simulink model "V2x_TC_RoadWorksWarning_cs"  (Simulink 7.6)
settings: Solver=ode5  SolverMode=SingleTasking  StartTime=0.0  StopTime=22  FixedStep=1/100  RelTol=1e-3
PreLoadFcn:
  | check_NUTS_defined;
  | 
  | assignin('base',['model_closed_' bdroot],false);addpath('../includes_V2x');
InitFcn:
  | PreScanExperimentInitFcn(bdroot)
StopFcn:
  | PreScanExperimentStopFcn(bdroot)
undefined symbols (not defined in the file or in its folder): acceleration, actorID, actorType, DiscIntNeverNeededParam, GPS_alt, GPS_lat, GPS_long, intmax, lightBarInUse, messageID, Newton, publicActorType, Raphson, rotZ, sireneInUse, trafficLightPriority, turnAdvice, velocity, x_ini_VRML, y_ini_VRML, yawRate, z_ini_VRML
libraries: simulink x62, PreScanSimulinkLibrary x45, PreScanUsefulBlocks x20, simulink_extras x1
(part 5 of 15)

== system V2x_TC_RoadWorksWarning_cs/STATE_Toyota_Yaris_1 ==
  State : Inport  SampleTime=TimeStep
  Acceleration : Subsystem  mask(ToSensorWorld){PortName=Acceleration_Toyota_Yaris_1, PortIndex=499, PortWidth=3, TimeStep=1/100}
      mask prompts: PortName "Port Name [-]"; PortIndex "Index [-]"; PortWidth "Port Dimensions [-]"; TimeStep "Upload Period [s]"
      mask help: Sends signal(s) from Simulink to the Sensor World
      mask Help:
      | Sends signal(s) from Simulink to the Sensor World.
      | 
      | - Port Name [-]: specifies the name of the output terminal of the Simulink block.
      | - Index [-]: specifies the start position in the output vector sent to the Sensor World
      | - Port dimensions [-]: specifies the width (number of scalars) of the output port
      | - Upload period [s]: specifies the upload period (in seconds) of the port.
      mask Display: port_label('input', 1, PortName)
  Demux : Demux  Outputs=5
  Position : Subsystem  mask(ToSensorWorld){PortName=Position_Toyota_Yaris_1, PortIndex=493, PortWidth=3, TimeStep=1/100}
      mask prompts: PortName "Port Name [-]"; PortIndex "Index [-]"; PortWidth "Port Dimensions [-]"; TimeStep "Upload Period [s]"
      mask help: Sends signal(s) from Simulink to the Sensor World
      mask Help:
      | Sends signal(s) from Simulink to the Sensor World.
      | 
      | - Port Name [-]: specifies the name of the output terminal of the Simulink block.
      | - Index [-]: specifies the start position in the output vector sent to the Sensor World
      | - Port dimensions [-]: specifies the width (number of scalars) of the output port
      | - Upload period [s]: specifies the upload period (in seconds) of the port.
      mask Display: port_label('input', 1, PortName)
  RPY : Subsystem  mask(ToSensorWorld){PortName=RPY_Toyota_Yaris_1, PortIndex=502, PortWidth=3, TimeStep=1/100}
      mask prompts: PortName "Port Name [-]"; PortIndex "Index [-]"; PortWidth "Port Dimensions [-]"; TimeStep "Upload Period [s]"
      mask help: Sends signal(s) from Simulink to the Sensor World
      mask Help:
      | Sends signal(s) from Simulink to the Sensor World.
      | 
      | - Port Name [-]: specifies the name of the output terminal of the Simulink block.
      | - Index [-]: specifies the start position in the output vector sent to the Sensor World
      | - Port dimensions [-]: specifies the width (number of scalars) of the output port
      | - Upload period [s]: specifies the upload period (in seconds) of the port.
      mask Display: port_label('input', 1, PortName)
  Velocity : Subsystem  mask(ToSensorWorld){PortName=Velocity_Toyota_Yaris_1, PortIndex=496, PortWidth=3, TimeStep=1/100}
      mask prompts: PortName "Port Name [-]"; PortIndex "Index [-]"; PortWidth "Port Dimensions [-]"; TimeStep "Upload Period [s]"
      mask help: Sends signal(s) from Simulink to the Sensor World
      mask Help:
      | Sends signal(s) from Simulink to the Sensor World.
      | 
      | - Port Name [-]: specifies the name of the output terminal of the Simulink block.
      | - Index [-]: specifies the start position in the output vector sent to the Sensor World
      | - Port dimensions [-]: specifies the width (number of scalars) of the output port
      | - Upload period [s]: specifies the upload period (in seconds) of the port.
      mask Display: port_label('input', 1, PortName)
  iniBODYAngles wrtVEH [rad] : Constant  Value=[Roll0 Pitch0 0]  SampleTime=TimeStep
  iniVEHAngles wrtGLOB [rad] : Constant  Value=[0 0 Yaw0]  SampleTime=TimeStep
  iniVEHposition wrtGLOB [m] : Constant  Value=[x0 y0 z0]  SampleTime=TimeStep
  state2FullState : PreScanSimulinkLibrary/state2FullState  SystemSampleTime=-1
  velRPY : Subsystem  mask(ToSensorWorld){PortName=velRPY_Toyota_Yaris_1, PortIndex=505, PortWidth=3, TimeStep=1/100}
      mask prompts: PortName "Port Name [-]"; PortIndex "Index [-]"; PortWidth "Port Dimensions [-]"; TimeStep "Upload Period [s]"
      mask help: Sends signal(s) from Simulink to the Sensor World
      mask Help:
      | Sends signal(s) from Simulink to the Sensor World.
      | 
      | - Port Name [-]: specifies the name of the output terminal of the Simulink block.
      | - Index [-]: specifies the start position in the output vector sent to the Sensor World
      | - Port dimensions [-]: specifies the width (number of scalars) of the output port
      | - Upload period [s]: specifies the upload period (in seconds) of the port.
      mask Display: port_label('input', 1, PortName)
  wires:
  Demux/5 -> velRPY/1(Y)
  Demux/4 -> RPY/1(Y)
  Demux/3 -> Acceleration/1(Y)
  Demux/2 -> Velocity/1(Y)
  Demux/1 -> Position/1(Y)
  State/1 -> state2FullState/1
  state2FullState/1 -> Demux/1
  iniVEHposition wrtGLOB [m]/1 -> state2FullState/2
  iniBODYAngles wrtVEH [rad]/1 -> state2FullState/3
  iniVEHAngles wrtGLOB [rad]/1 -> state2FullState/4

== system V2x_TC_RoadWorksWarning_cs/SetActuators ==
  Bus Creator : BusCreator  Inputs=1
  Data Store Read : DataStoreRead  DataStoreName=SimCoreActuators  SampleTime=TimeStep
  SendActuators : M-S-Function  FunctionName=SendActuatorsBlock  Parameters=TimeStep NrOfActuators
  dummy : Outport
  tag scope: SimCoreInfo=global
  wires:
  Bus Creator/1 -> [SimCoreInfo]  "SimCore"
  SendActuators/2 -> Bus Creator/1  "ComputationTime[s]"
  SendActuators/1 -> dummy/1
  Data Store Read/1 -> SendActuators/1

== system V2x_TC_RoadWorksWarning_cs/SimCoreInfo ==
  Bus Selector : BusSelector  OutputSignals=ComputationTime[s]
  Time : Scope
      settings: TimeRange=10  YMin=0  YMax=0.5  SaveName=ComputationTime
  wires:
  Bus Selector/1 -> Time/1  "<ComputationTime[s]>"
  [SimCoreInfo] -> Bus Selector/1

== system V2x_TC_RoadWorksWarning_cs/Sphere_1 ==
  SELF_Sphere_1 : Inport  Tag=SELFInport
  Dynamics_Empty : Subsystem  Tag=Dynamics_Empty
      mask Display: port_label('output', 1, 'State');text(0,0,' Empty\n')
  Gain : Gain  Gain=[1 1 1 1 1 1 0 0 1]
  SELF_Demux : Subsystem  Tag=DemuxSELF
      settings: DeleteFcn=PreScanBlockDeleteFcn;
      mask Type: PreScan Demux Block:
      mask Display:
      | port_label('output',1,'x [m]')
      | port_label('output',2,'y [m]')
      | port_label('output',3,'z [m]','texmode','on')
      | port_label('output',4,'Rotx [deg]','texmode','on')
      | port_label('output',5,'Roty [deg]','texmode','on')
      | port_label('output',6,'Rotz [deg]','texmode','on')
      | port_label('output',7,'GPS lat [deg]','texmode','on')
      | port_label('output',8,'GPS long [deg]','texmode','on')
      | port_label('output',9,'GPS alt [m]','texmode','on')
      | port_label('output',10,'Velocity [m/s]')
      | port_label('output',11,'Heading [deg]')
      | port_label('output',12,'Yaw rate [deg/s]')
  Terminator_{1..13} (13 alike) : Terminator
  STATE_Sphere_1 : Outport  Tag=StateOutport
  wires:
  [state] -> Gain/1
  SELF_Sphere_1/1 -> SELF_Demux/1(SELF)
  Gain/1 -> STATE_Sphere_1/1
  Dynamics_Empty/1(State) -> Terminator_1/1
  SELF_Demux/1(x [m]) -> Terminator_2/1
  SELF_Demux/2(y [m]) -> Terminator_3/1
  SELF_Demux/3(z [m]) -> Terminator_4/1
  SELF_Demux/4(rotx [deg]) -> Terminator_5/1
  SELF_Demux/5(roty [deg]) -> Terminator_6/1
  SELF_Demux/6(rotz [deg]) -> Terminator_7/1
  SELF_Demux/7(GPS lat [deg]) -> Terminator_8/1
  SELF_Demux/8(GPS long [deg]) -> Terminator_9/1
  SELF_Demux/9(GPS alt [m]) -> Terminator_10/1
  SELF_Demux/10(velocity [m/s]) -> Terminator_11/1
  SELF_Demux/11(heading [deg]) -> Terminator_12/1
  SELF_Demux/12(yaw rate [deg/s]) -> Terminator_13/1

== system V2x_TC_RoadWorksWarning_cs/Toyota_Previa_1 ==
  SELF_Toyota_Previa_1 : Inport  Tag=SELFInport
  Dynamics_Empty : Subsystem  Tag=Dynamics_Empty
      mask Display: port_label('output', 1, 'State');text(0,0,' Empty\n')
  SELF_Demux : Subsystem  Tag=DemuxSELF
      settings: DeleteFcn=PreScanBlockDeleteFcn;
      mask Type: PreScan Demux Block:
      mask Display:
      | port_label('output',1,'x [m]')
      | port_label('output',2,'y [m]')
      | port_label('output',3,'z [m]','texmode','on')
      | port_label('output',4,'Rotx [deg]','texmode','on')
      | port_label('output',5,'Roty [deg]','texmode','on')
      | port_label('output',6,'Rotz [deg]','texmode','on')
      | port_label('output',7,'GPS lat [deg]','texmode','on')
      | port_label('output',8,'GPS long [deg]','texmode','on')
      | port_label('output',9,'GPS alt [m]','texmode','on')
      | port_label('output',10,'Velocity [m/s]')
      | port_label('output',11,'Heading [deg]')
      | port_label('output',12,'Yaw rate [deg/s]')
  Terminator_{1..12} (12 alike) : Terminator
  STATE_Toyota_Previa_1 : Outport  Tag=StateOutport
  wires:
  SELF_Toyota_Previa_1/1 -> SELF_Demux/1(SELF)
  Dynamics_Empty/1(State) -> STATE_Toyota_Previa_1/1
  SELF_Demux/1(x [m]) -> Terminator_1/1
  SELF_Demux/2(y [m]) -> Terminator_2/1
  SELF_Demux/3(z [m]) -> Terminator_3/1
  SELF_Demux/4(rotx [deg]) -> Terminator_4/1
  SELF_Demux/5(roty [deg]) -> Terminator_5/1
  SELF_Demux/6(rotz [deg]) -> Terminator_6/1
  SELF_Demux/7(GPS lat [deg]) -> Terminator_7/1
  SELF_Demux/8(GPS long [deg]) -> Terminator_8/1
  SELF_Demux/9(GPS alt [m]) -> Terminator_9/1
  SELF_Demux/10(velocity [m/s]) -> Terminator_10/1
  SELF_Demux/11(heading [deg]) -> Terminator_11/1
  SELF_Demux/12(yaw rate [deg/s]) -> Terminator_12/1

== system V2x_TC_RoadWorksWarning_cs/Toyota_Yaris_1 ==
  SELF_Toyota_Yaris_1 : Inport  Tag=SELFInport
  Dynamics_Empty : Subsystem  Tag=Dynamics_Empty
      mask Display: port_label('output', 1, 'State');text(0,0,' Empty\n')
  SELF_Demux : Subsystem  Tag=DemuxSELF
      settings: DeleteFcn=PreScanBlockDeleteFcn;
      mask Type: PreScan Demux Block:
      mask Display:
      | port_label('output',1,'x [m]')
      | port_label('output',2,'y [m]')
      | port_label('output',3,'z [m]','texmode','on')
      | port_label('output',4,'Rotx [deg]','texmode','on')
      | port_label('output',5,'Roty [deg]','texmode','on')
      | port_label('output',6,'Rotz [deg]','texmode','on')
      | port_label('output',7,'GPS lat [deg]','texmode','on')
      | port_label('output',8,'GPS long [deg]','texmode','on')
      | port_label('output',9,'GPS alt [m]','texmode','on')
      | port_label('output',10,'Velocity [m/s]')
      | port_label('output',11,'Heading [deg]')
      | port_label('output',12,'Yaw rate [deg/s]')
  Terminator_{1..12} (12 alike) : Terminator
  STATE_Toyota_Yaris_1 : Outport  Tag=StateOutport
  wires:
  SELF_Toyota_Yaris_1/1 -> SELF_Demux/1(SELF)
  Dynamics_Empty/1(State) -> STATE_Toyota_Yaris_1/1
  SELF_Demux/1(x [m]) -> Terminator_1/1
  SELF_Demux/2(y [m]) -> Terminator_2/1
  SELF_Demux/3(z [m]) -> Terminator_3/1
  SELF_Demux/4(rotx [deg]) -> Terminator_4/1
  SELF_Demux/5(roty [deg]) -> Terminator_5/1
  SELF_Demux/6(rotz [deg]) -> Terminator_6/1
  SELF_Demux/7(GPS lat [deg]) -> Terminator_7/1
  SELF_Demux/8(GPS long [deg]) -> Terminator_8/1
  SELF_Demux/9(GPS alt [m]) -> Terminator_9/1
  SELF_Demux/10(velocity [m/s]) -> Terminator_10/1
  SELF_Demux/11(heading [deg]) -> Terminator_11/1
  SELF_Demux/12(yaw rate [deg/s]) -> Terminator_12/1

== system V2x_TC_RoadWorksWarning_cs/TrafficSignPoleD_1 ==
  Antenna_Tx_6_BusAssignment : BusAssignment  Tag=BusAntennaT  AssignedSignals=warning,messageID,actorID,actorType,trafficLightPriority,GPS_lat,GPS_long,GPS_alt,rotZ
  Antenna_Tx_6_Mux : Subsystem  Tag=MuxAntennaT  mask(PreScan Mux Block:){Channel=1, TimeStep=1/100}
      mask prompts: TimeStep "Time Step [s]"
      settings: DeleteFcn=PreScanBlockDeleteFcn;
      mask Display:
      | fprintf('Channel: %d',Channel)
      | port_label('input', 1, 'Warning signal ID')
      | port_label('input', 2, 'Maneuvre ID')
      | port_label('input', 3, 'Vehicle Type')
      | port_label('input', 4, 'Braking Capacity')
      | port_label('input', 5, 'Vehicle Size')
      | port_label('input', 6, 'spare')
  CAM generation time step : Constant  Value=0.1
  CAM repetition time step : Constant  Value=0.05
  Cartesian to GPS : Subsystem
  Green light : Constant  Value=0
  Ground_{1..5} (5 alike) : Ground
  Infrastructure CAM generator : Subsystem
  Orange light : Constant  Value=0
  Red light : Constant  Value=0
  X : Constant  Value=200
  Y : Constant  Value=-6
  Z : Constant  Value=0
  actorID : Constant  Value=9
  actorType : Constant  Value=14
  messageID : Constant  Value=2
  rotZ : Constant  Value=180
  warning : Constant  Value=0
  Antenna_Tx_6 : Outport  Tag=AntennaTOutport
  wires:
  CAM generation time step/1 -> Infrastructure CAM generator/1(CAM gen time step)
  CAM repetition time step/1 -> Infrastructure CAM generator/2(CAM rep time step)
  warning/1 -> Infrastructure CAM generator/3(warning)
  messageID/1 -> Infrastructure CAM generator/4(messageID)
  actorID/1 -> Infrastructure CAM generator/5(actorID)
  actorType/1 -> Infrastructure CAM generator/6(actorType)
  Red light/1 -> Infrastructure CAM generator/7(Red light)
  Orange light/1 -> Infrastructure CAM generator/8(Orange light)
  Green light/1 -> Infrastructure CAM generator/9(Green light)
  rotZ/1 -> Infrastructure CAM generator/13(rotZ)
  Cartesian to GPS/1(lat) -> Infrastructure CAM generator/10(GPS_lat)
  Cartesian to GPS/2(long) -> Infrastructure CAM generator/11(GPS_long)
  Cartesian to GPS/3(alt) -> Infrastructure CAM generator/12(GPS_alt)
  X/1 -> Cartesian to GPS/1(X)
  Y/1 -> Cartesian to GPS/2(Y)
  Z/1 -> Cartesian to GPS/3(Z)
  Antenna_Tx_6_Mux/2(Out2) -> [Antenna_Tx_6]
  [Antenna_Tx_6] -> Antenna_Tx_6_BusAssignment/1
  Antenna_Tx_6_BusAssignment/1 -> Antenna_Tx_6_Mux/6(spare)
  Antenna_Tx_6_Mux/1(Out1) -> Antenna_Tx_6/1
  Infrastructure CAM generator/9(CAM_rotZ) -> Antenna_Tx_6_BusAssignment/10
  Infrastructure CAM generator/8(CAM_GPSalt) -> Antenna_Tx_6_BusAssignment/9
  Infrastructure CAM generator/7(CAM_GPSlong) -> Antenna_Tx_6_BusAssignment/8
  Infrastructure CAM generator/6(CAM_GPSlat) -> Antenna_Tx_6_BusAssignment/7
  Infrastructure CAM generator/5(CAM_trafficLightPriority) -> Antenna_Tx_6_BusAssignment/6
  Infrastructure CAM generator/4(CAM_vehicleType) -> Antenna_Tx_6_BusAssignment/5
  Infrastructure CAM generator/3(CAM_vehicleID) -> Antenna_Tx_6_BusAssignment/4
  Infrastructure CAM generator/2(CAM_messageID) -> Antenna_Tx_6_BusAssignment/3
  Infrastructure CAM generator/1(CAM_warning) -> Antenna_Tx_6_BusAssignment/2
  Ground_1/1 -> Antenna_Tx_6_Mux/1(warning_signal_id)
  Ground_2/1 -> Antenna_Tx_6_Mux/2(maneuvre_id)
  Ground_3/1 -> Antenna_Tx_6_Mux/3(vehicle_type)
  Ground_4/1 -> Antenna_Tx_6_Mux/4(brakingcapacity)
  Ground_5/1 -> Antenna_Tx_6_Mux/5(vehicle_size)

== system V2x_TC_RoadWorksWarning_cs/TrafficSignPoleD_3 ==
  Antenna_Tx_4_BusAssignment : BusAssignment  Tag=BusAntennaT  AssignedSignals=actorID,warning,actorType,lightBarInUse,sireneInUse,trafficLightPriority,GPS_lat,GPS_long,GPS_alt
  Antenna_Tx_4_Mux : Subsystem  Tag=MuxAntennaT  mask(PreScan Mux Block:){Channel=1, TimeStep=1/100}
      mask prompts: TimeStep "Time Step [s]"
      settings: DeleteFcn=PreScanBlockDeleteFcn;
      mask Display:
      | fprintf('Channel: %d',Channel)
      | port_label('input', 1, 'Warning signal ID')
      | port_label('input', 2, 'Maneuvre ID')
      | port_label('input', 3, 'Vehicle Type')
      | port_label('input', 4, 'Braking Capacity')
      | port_label('input', 5, 'Vehicle Size')
      | port_label('input', 6, 'spare')
  CAM generation time step : Constant  Value=0.1
  CAM repetition time step : Constant  Value=0.05
  Cartesian to GPS : Subsystem
  Ground_{1..5} (5 alike) : Ground
  ITS actorID : Constant  Value=161
  ITS actorType : Constant  Value=25
  Infrastructure CAM generator ITS road : Subsystem
  Red light : Constant  Value=0
  Red light1 : Constant  Value=0
  Red light2 : Constant  Value=0
  X : Constant  Value=220
  Y : Constant  Value=-6.5
  Z : Constant  Value=0
  actorType : Constant
  Antenna_Tx_4 : Outport  Tag=AntennaTOutport
  wires:
  CAM generation time step/1 -> Infrastructure CAM generator ITS road/1(CAM gen time step)
  CAM repetition time step/1 -> Infrastructure CAM generator ITS road/2(CAM rep time step)
  ITS actorID/1 -> Infrastructure CAM generator ITS road/3(ITS actorID)
  ITS actorType/1 -> Infrastructure CAM generator ITS road/4(ITS actorType)
  [transmission] -> Infrastructure CAM generator ITS road/5(warning)
  actorType/1 -> Infrastructure CAM generator ITS road/6(actorType)
  Red light/1 -> Infrastructure CAM generator ITS road/7(lightBarInUse)
  Red light1/1 -> Infrastructure CAM generator ITS road/9(trafficLightPriority)
  X/1 -> Cartesian to GPS/1(X)
  Y/1 -> Cartesian to GPS/2(Y)
  Z/1 -> Cartesian to GPS/3(Z)
  Red light2/1 -> Infrastructure CAM generator ITS road/8(sireneInUse)
  Cartesian to GPS/1(lat) -> Infrastructure CAM generator ITS road/10(GPS_lat)
  Cartesian to GPS/2(long) -> Infrastructure CAM generator ITS road/11(GPS_long)
  Cartesian to GPS/3(alt) -> Infrastructure CAM generator ITS road/12(GPS_alt)
  Antenna_Tx_4_Mux/2(Out2) -> [Antenna_Tx_4]
  [Antenna_Tx_4] -> Antenna_Tx_4_BusAssignment/1
  Antenna_Tx_4_BusAssignment/1 -> Antenna_Tx_4_Mux/6(spare)
  Antenna_Tx_4_Mux/1(Out1) -> Antenna_Tx_4/1
  Infrastructure CAM generator ITS road/1(CAM_actorID) -> Antenna_Tx_4_BusAssignment/2
  Infrastructure CAM generator ITS road/2(CAM_warning) -> Antenna_Tx_4_BusAssignment/3
  Infrastructure CAM generator ITS road/3(CAM_actorType) -> Antenna_Tx_4_BusAssignment/4
  Infrastructure CAM generator ITS road/4(CAM_lightBarInUse) -> Antenna_Tx_4_BusAssignment/5
  Infrastructure CAM generator ITS road/5(CAM_sireneInUse) -> Antenna_Tx_4_BusAssignment/6
  Infrastructure CAM generator ITS road/6(CAM_trafficLightPriority) -> Antenna_Tx_4_BusAssignment/7
  Infrastructure CAM generator ITS road/7(CAM_GPS_lat) -> Antenna_Tx_4_BusAssignment/8
  Infrastructure CAM generator ITS road/8(CAM_GPS_long) -> Antenna_Tx_4_BusAssignment/9
  Infrastructure CAM generator ITS road/9(CAM_GPS_alt) -> Antenna_Tx_4_BusAssignment/10
  Ground_1/1 -> Antenna_Tx_4_Mux/1(warning_signal_id)
  Ground_2/1 -> Antenna_Tx_4_Mux/2(maneuvre_id)
  Ground_3/1 -> Antenna_Tx_4_Mux/3(vehicle_type)
  Ground_4/1 -> Antenna_Tx_4_Mux/4(brakingcapacity)
  Ground_5/1 -> Antenna_Tx_4_Mux/5(vehicle_size)

== system V2x_TC_RoadWorksWarning_cs/TrafficSignPoleD_6 ==
  Antenna_Tx_5_BusAssignment : BusAssignment  Tag=BusAntennaT  AssignedSignals=warning,messageID,actorID,actorType,trafficLightPriority,GPS_lat,GPS_long,GPS_alt,rotZ
  Antenna_Tx_5_Mux : Subsystem  Tag=MuxAntennaT  mask(PreScan Mux Block:){Channel=1, TimeStep=1/100}
      mask prompts: TimeStep "Time Step [s]"
      settings: DeleteFcn=PreScanBlockDeleteFcn;
      mask Display:
      | fprintf('Channel: %d',Channel)
      | port_label('input', 1, 'Warning signal ID')
      | port_label('input', 2, 'Maneuvre ID')
      | port_label('input', 3, 'Vehicle Type')
      | port_label('input', 4, 'Braking Capacity')
      | port_label('input', 5, 'Vehicle Size')
      | port_label('input', 6, 'spare')
  CAM generation time step : Constant  Value=0.1
  CAM repetition time step : Constant  Value=0.05
  Cartesian to GPS : Subsystem
  Green light : Constant  Value=0
  Ground_{1..5} (5 alike) : Ground
  Infrastructure CAM generator : Subsystem
  Orange light : Constant  Value=0
  Red light : Constant  Value=0
  X : Constant  Value=110
  Y : Constant  Value=-6.5
  Z : Constant  Value=0
  actorID : Constant  Value=8
  actorType : Constant  Value=14
  messageID : Constant
  rotZ : Constant  Value=180
  warning : Constant  Value=0
  Antenna_Tx_5 : Outport  Tag=AntennaTOutport
  wires:
  CAM generation time step/1 -> Infrastructure CAM generator/1(CAM gen time step)
  CAM repetition time step/1 -> Infrastructure CAM generator/2(CAM rep time step)
  warning/1 -> Infrastructure CAM generator/3(warning)
  messageID/1 -> Infrastructure CAM generator/4(messageID)
  actorID/1 -> Infrastructure CAM generator/5(actorID)
  actorType/1 -> Infrastructure CAM generator/6(actorType)
  Red light/1 -> Infrastructure CAM generator/7(Red light)
  Orange light/1 -> Infrastructure CAM generator/8(Orange light)
  Green light/1 -> Infrastructure CAM generator/9(Green light)
  rotZ/1 -> Infrastructure CAM generator/13(rotZ)
  Cartesian to GPS/1(lat) -> Infrastructure CAM generator/10(GPS_lat)
  Cartesian to GPS/2(long) -> Infrastructure CAM generator/11(GPS_long)
  Cartesian to GPS/3(alt) -> Infrastructure CAM generator/12(GPS_alt)
  X/1 -> Cartesian to GPS/1(X)
  Y/1 -> Cartesian to GPS/2(Y)
  Z/1 -> Cartesian to GPS/3(Z)
  Antenna_Tx_5_Mux/2(Out2) -> [Antenna_Tx_5]
  [Antenna_Tx_5] -> Antenna_Tx_5_BusAssignment/1
  Antenna_Tx_5_BusAssignment/1 -> Antenna_Tx_5_Mux/6(spare)
  Antenna_Tx_5_Mux/1(Out1) -> Antenna_Tx_5/1
  Infrastructure CAM generator/9(CAM_rotZ) -> Antenna_Tx_5_BusAssignment/10
  Infrastructure CAM generator/8(CAM_GPSalt) -> Antenna_Tx_5_BusAssignment/9
  Infrastructure CAM generator/7(CAM_GPSlong) -> Antenna_Tx_5_BusAssignment/8
  Infrastructure CAM generator/6(CAM_GPSlat) -> Antenna_Tx_5_BusAssignment/7
  Infrastructure CAM generator/5(CAM_trafficLightPriority) -> Antenna_Tx_5_BusAssignment/6
  Infrastructure CAM generator/4(CAM_vehicleType) -> Antenna_Tx_5_BusAssignment/5
  Infrastructure CAM generator/3(CAM_vehicleID) -> Antenna_Tx_5_BusAssignment/4
  Infrastructure CAM generator/2(CAM_messageID) -> Antenna_Tx_5_BusAssignment/3
  Infrastructure CAM generator/1(CAM_warning) -> Antenna_Tx_5_BusAssignment/2
  Ground_1/1 -> Antenna_Tx_5_Mux/1(warning_signal_id)
  Ground_2/1 -> Antenna_Tx_5_Mux/2(maneuvre_id)
  Ground_3/1 -> Antenna_Tx_5_Mux/3(vehicle_type)
  Ground_4/1 -> Antenna_Tx_5_Mux/4(brakingcapacity)
  Ground_5/1 -> Antenna_Tx_5_Mux/5(vehicle_size)

== system V2x_TC_RoadWorksWarning_cs/TrafficSignPoleNL_1 ==
  Antenna_Tx_2_BusAssignment : BusAssignment  Tag=BusAntennaT  AssignedSignals=warning,messageID,actorID,actorType,trafficLightPriority,GPS_lat,GPS_long,GPS_alt,rotZ
  Antenna_Tx_2_Mux : Subsystem  Tag=MuxAntennaT  mask(PreScan Mux Block:){Channel=1, TimeStep=1/100}
      mask prompts: TimeStep "Time Step [s]"
      settings: DeleteFcn=PreScanBlockDeleteFcn;
      mask Display:
      | fprintf('Channel: %d',Channel)
      | port_label('input', 1, 'Warning signal ID')
      | port_label('input', 2, 'Maneuvre ID')
      | port_label('input', 3, 'Vehicle Type')
      | port_label('input', 4, 'Braking Capacity')
      | port_label('input', 5, 'Vehicle Size')
      | port_label('input', 6, 'spare')
  CAM generation time step : Constant  Value=0.1
  CAM repetition time step : Constant  Value=0.05
  Cartesian to GPS : Subsystem
  Green light : Constant  Value=0
  Ground_{1..5} (5 alike) : Ground
  Infrastructure CAM generator : Subsystem
  Orange light : Constant  Value=0
  Red light : Constant  Value=0
  X : Constant  Value=122
  Y : Constant  Value=-6
  Z : Constant  Value=0
  actorID : Constant  Value=7
  actorType : Constant  Value=14
  messageID : Constant  Value=1060
  rotZ : Constant  Value=180
  warning : Constant  Value=0
  Antenna_Tx_2 : Outport  Tag=AntennaTOutport
  wires:
  CAM generation time step/1 -> Infrastructure CAM generator/1(CAM gen time step)
  CAM repetition time step/1 -> Infrastructure CAM generator/2(CAM rep time step)
  warning/1 -> Infrastructure CAM generator/3(warning)
  messageID/1 -> Infrastructure CAM generator/4(messageID)
  actorID/1 -> Infrastructure CAM generator/5(actorID)
  actorType/1 -> Infrastructure CAM generator/6(actorType)
  Red light/1 -> Infrastructure CAM generator/7(Red light)
  Orange light/1 -> Infrastructure CAM generator/8(Orange light)
  Green light/1 -> Infrastructure CAM generator/9(Green light)
  rotZ/1 -> Infrastructure CAM generator/13(rotZ)
  Cartesian to GPS/1(lat) -> Infrastructure CAM generator/10(GPS_lat)
  Cartesian to GPS/2(long) -> Infrastructure CAM generator/11(GPS_long)
  Cartesian to GPS/3(alt) -> Infrastructure CAM generator/12(GPS_alt)
  X/1 -> Cartesian to GPS/1(X)
  Y/1 -> Cartesian to GPS/2(Y)
  Z/1 -> Cartesian to GPS/3(Z)
  Antenna_Tx_2_Mux/2(Out2) -> [Antenna_Tx_2]
  [Antenna_Tx_2] -> Antenna_Tx_2_BusAssignment/1
  Antenna_Tx_2_BusAssignment/1 -> Antenna_Tx_2_Mux/6(spare)
  Antenna_Tx_2_Mux/1(Out1) -> Antenna_Tx_2/1
  Infrastructure CAM generator/9(CAM_rotZ) -> Antenna_Tx_2_BusAssignment/10
  Infrastructure CAM generator/8(CAM_GPSalt) -> Antenna_Tx_2_BusAssignment/9
  Infrastructure CAM generator/7(CAM_GPSlong) -> Antenna_Tx_2_BusAssignment/8
  Infrastructure CAM generator/6(CAM_GPSlat) -> Antenna_Tx_2_BusAssignment/7
  Infrastructure CAM generator/5(CAM_trafficLightPriority) -> Antenna_Tx_2_BusAssignment/6
  Infrastructure CAM generator/4(CAM_vehicleType) -> Antenna_Tx_2_BusAssignment/5
  Infrastructure CAM generator/3(CAM_vehicleID) -> Antenna_Tx_2_BusAssignment/4
  Infrastructure CAM generator/2(CAM_messageID) -> Antenna_Tx_2_BusAssignment/3
  Infrastructure CAM generator/1(CAM_warning) -> Antenna_Tx_2_BusAssignment/2
  Ground_1/1 -> Antenna_Tx_2_Mux/1(warning_signal_id)
  Ground_2/1 -> Antenna_Tx_2_Mux/2(maneuvre_id)
  Ground_3/1 -> Antenna_Tx_2_Mux/3(vehicle_type)
  Ground_4/1 -> Antenna_Tx_2_Mux/4(brakingcapacity)
  Ground_5/1 -> Antenna_Tx_2_Mux/5(vehicle_size)

== system V2x_TC_RoadWorksWarning_cs/TrafficSignPoleNL_3 ==
  Antenna_Tx_3_BusAssignment : BusAssignment  Tag=BusAntennaT  AssignedSignals=warning,messageID,actorID,actorType,trafficLightPriority,GPS_lat,GPS_long,GPS_alt,rotZ
  Antenna_Tx_3_Mux : Subsystem  Tag=MuxAntennaT  mask(PreScan Mux Block:){Channel=1, TimeStep=1/100}
      mask prompts: TimeStep "Time Step [s]"
      settings: DeleteFcn=PreScanBlockDeleteFcn;
      mask Display:
      | fprintf('Channel: %d',Channel)
      | port_label('input', 1, 'Warning signal ID')
      | port_label('input', 2, 'Maneuvre ID')
      | port_label('input', 3, 'Vehicle Type')
      | port_label('input', 4, 'Braking Capacity')
      | port_label('input', 5, 'Vehicle Size')
      | port_label('input', 6, 'spare')
  CAM generation time step : Constant  Value=0.1
  CAM repetition time step : Constant  Value=0.05
  Cartesian to GPS : Subsystem
  Green light : Constant  Value=0
  Ground_{1..5} (5 alike) : Ground
  Infrastructure CAM generator : Subsystem
  Orange light : Constant  Value=0
  Red light : Constant  Value=0
  X : Constant  Value=270
  Y : Constant  Value=-6
  Z : Constant  Value=0
  actorID : Constant  Value=7
  actorType : Constant  Value=14
  messageID : Constant  Value=1060
  rotZ : Constant  Value=180
  warning : Constant  Value=0
  Antenna_Tx_3 : Outport  Tag=AntennaTOutport
  wires:
  CAM generation time step/1 -> Infrastructure CAM generator/1(CAM gen time step)
  CAM repetition time step/1 -> Infrastructure CAM generator/2(CAM rep time step)
  warning/1 -> Infrastructure CAM generator/3(warning)
  messageID/1 -> Infrastructure CAM generator/4(messageID)
  actorID/1 -> Infrastructure CAM generator/5(actorID)
  actorType/1 -> Infrastructure CAM generator/6(actorType)
  Red light/1 -> Infrastructure CAM generator/7(Red light)
  Orange light/1 -> Infrastructure CAM generator/8(Orange light)
  Green light/1 -> Infrastructure CAM generator/9(Green light)
  rotZ/1 -> Infrastructure CAM generator/13(rotZ)
  Cartesian to GPS/1(lat) -> Infrastructure CAM generator/10(GPS_lat)
  Cartesian to GPS/2(long) -> Infrastructure CAM generator/11(GPS_long)
  Cartesian to GPS/3(alt) -> Infrastructure CAM generator/12(GPS_alt)
  X/1 -> Cartesian to GPS/1(X)
  Y/1 -> Cartesian to GPS/2(Y)
  Z/1 -> Cartesian to GPS/3(Z)
  Antenna_Tx_3_Mux/2(Out2) -> [Antenna_Tx_3]
  [Antenna_Tx_3] -> Antenna_Tx_3_BusAssignment/1
  Antenna_Tx_3_BusAssignment/1 -> Antenna_Tx_3_Mux/6(spare)
  Antenna_Tx_3_Mux/1(Out1) -> Antenna_Tx_3/1
  Infrastructure CAM generator/1(CAM_warning) -> Antenna_Tx_3_BusAssignment/2
  Infrastructure CAM generator/2(CAM_messageID) -> Antenna_Tx_3_BusAssignment/3
  Infrastructure CAM generator/3(CAM_vehicleID) -> Antenna_Tx_3_BusAssignment/4
  Infrastructure CAM generator/4(CAM_vehicleType) -> Antenna_Tx_3_BusAssignment/5
  Infrastructure CAM generator/5(CAM_trafficLightPriority) -> Antenna_Tx_3_BusAssignment/6
  Infrastructure CAM generator/6(CAM_GPSlat) -> Antenna_Tx_3_BusAssignment/7
  Infrastructure CAM generator/7(CAM_GPSlong) -> Antenna_Tx_3_BusAssignment/8
  Infrastructure CAM generator/8(CAM_GPSalt) -> Antenna_Tx_3_BusAssignment/9
  Infrastructure CAM generator/9(CAM_rotZ) -> Antenna_Tx_3_BusAssignment/10
  Ground_1/1 -> Antenna_Tx_3_Mux/1(warning_signal_id)
  Ground_2/1 -> Antenna_Tx_3_Mux/2(maneuvre_id)
  Ground_3/1 -> Antenna_Tx_3_Mux/3(vehicle_type)
  Ground_4/1 -> Antenna_Tx_3_Mux/4(brakingcapacity)
  Ground_5/1 -> Antenna_Tx_3_Mux/5(vehicle_size)

== system V2x_TC_RoadWorksWarning_cs/VISULightSource_Audi_A8_1 ==
  Lights : Inport
  Saturation{1..12} (12 alike) : Saturate  UpperLimit=1  LowerLimit=0
  SubDemuxVisuLightSource : Demux  Outputs=12
  conv_BrakeLightLeft : Subsystem
  conv_BrakeLightRight : Subsystem
  conv_FogLightFrontLeft : Subsystem
  conv_FogLightFrontRight : Subsystem
  conv_FogLightRearLeft : Subsystem
  conv_FogLightRearRight : Subsystem
  conv_IndicatorLightsLeft : Subsystem
  conv_IndicatorLightsRight : Subsystem
  conv_MainLightFrontLeft : Subsystem
  conv_MainLightFrontRight : Subsystem
  conv_MainLightRearLeft : Subsystem
  conv_MainLightRearRight : Subsystem
  visu_BrakeLightLeft_0 : Subsystem  mask(ToSensorWorld){PortName=visu_BrakeLightLeft_0, PortIndex=380, PortWidth=16, TimeStep=1/100}
      mask prompts: PortName "Port Name [-]"; PortIndex "Index [-]"; PortWidth "Port Dimensions [-]"; TimeStep "Upload Period [s]"
      mask help: Sends signal(s) from Simulink to the Sensor World
      mask Help:
      | Sends signal(s) from Simulink to the Sensor World.
      | 
      | - Port Name [-]: specifies the name of the output terminal of the Simulink block.
      | - Index [-]: specifies the start position in the output vector sent to the Sensor World
      | - Port dimensions [-]: specifies the width (number of scalars) of the output port
      | - Upload period [s]: specifies the upload period (in seconds) of the port.
      mask Display: port_label('input', 1, PortName)
  visu_BrakeLightRight_0 : Subsystem  mask(ToSensorWorld){PortName=visu_BrakeLightRight_0, PortIndex=396, PortWidth=16, TimeStep=1/100}
      mask prompts: PortName "Port Name [-]"; PortIndex "Index [-]"; PortWidth "Port Dimensions [-]"; TimeStep "Upload Period [s]"
      mask help: Sends signal(s) from Simulink to the Sensor World
      mask Help:
      | Sends signal(s) from Simulink to the Sensor World.
      | 
      | - Port Name [-]: specifies the name of the output terminal of the Simulink block.
      | - Index [-]: specifies the start position in the output vector sent to the Sensor World
      | - Port dimensions [-]: specifies the width (number of scalars) of the output port
      | - Upload period [s]: specifies the upload period (in seconds) of the port.
      mask Display: port_label('input', 1, PortName)
  visu_FogLightFrontLeft_0 : Subsystem  mask(ToSensorWorld){PortName=visu_FogLightFrontLeft_0, PortIndex=284, PortWidth=16, TimeStep=1/100}
      mask prompts: PortName "Port Name [-]"; PortIndex "Index [-]"; PortWidth "Port Dimensions [-]"; TimeStep "Upload Period [s]"
      mask help: Sends signal(s) from Simulink to the Sensor World
      mask Help:
      | Sends signal(s) from Simulink to the Sensor World.
      | 
      | - Port Name [-]: specifies the name of the output terminal of the Simulink block.
      | - Index [-]: specifies the start position in the output vector sent to the Sensor World
      | - Port dimensions [-]: specifies the width (number of scalars) of the output port
      | - Upload period [s]: specifies the upload period (in seconds) of the port.
      mask Display: port_label('input', 1, PortName)
  visu_FogLightFrontRight_0 : Subsystem  mask(ToSensorWorld){PortName=visu_FogLightFrontRight_0, PortIndex=300, PortWidth=16, TimeStep=1/100}
      mask prompts: PortName "Port Name [-]"; PortIndex "Index [-]"; PortWidth "Port Dimensions [-]"; TimeStep "Upload Period [s]"
      mask help: Sends signal(s) from Simulink to the Sensor World
      mask Help:
      | Sends signal(s) from Simulink to the Sensor World.
      | 
      | - Port Name [-]: specifies the name of the output terminal of the Simulink block.
      | - Index [-]: specifies the start position in the output vector sent to the Sensor World
      | - Port dimensions [-]: specifies the width (number of scalars) of the output port
      | - Upload period [s]: specifies the upload period (in seconds) of the port.
      mask Display: port_label('input', 1, PortName)
  visu_FogLightRearLeft_0 : Subsystem  mask(ToSensorWorld){PortName=visu_FogLightRearLeft_0, PortIndex=252, PortWidth=16, TimeStep=1/100}
      mask prompts: PortName "Port Name [-]"; PortIndex "Index [-]"; PortWidth "Port Dimensions [-]"; TimeStep "Upload Period [s]"
      mask help: Sends signal(s) from Simulink to the Sensor World
      mask Help:
      | Sends signal(s) from Simulink to the Sensor World.
      | 
      | - Port Name [-]: specifies the name of the output terminal of the Simulink block.
      | - Index [-]: specifies the start position in the output vector sent to the Sensor World
      | - Port dimensions [-]: specifies the width (number of scalars) of the output port
      | - Upload period [s]: specifies the upload period (in seconds) of the port.
      mask Display: port_label('input', 1, PortName)
  visu_FogLightRearRight_0 : Subsystem  mask(ToSensorWorld){PortName=visu_FogLightRearRight_0, PortIndex=268, PortWidth=16, TimeStep=1/100}
      mask prompts: PortName "Port Name [-]"; PortIndex "Index [-]"; PortWidth "Port Dimensions [-]"; TimeStep "Upload Period [s]"
      mask help: Sends signal(s) from Simulink to the Sensor World
      mask Help:
      | Sends signal(s) from Simulink to the Sensor World.
      | 
      | - Port Name [-]: specifies the name of the output terminal of the Simulink block.
      | - Index [-]: specifies the start position in the output vector sent to the Sensor World
      | - Port dimensions [-]: specifies the width (number of scalars) of the output port
      | - Upload period [s]: specifies the upload period (in seconds) of the port.
      mask Display: port_label('input', 1, PortName)
  visu_IndicatorLightsLeft_0 : Subsystem  mask(ToSensorWorld){PortName=visu_IndicatorLightsLeft_0, PortIndex=220, PortWidth=16, TimeStep=1/100}
      mask prompts: PortName "Port Name [-]"; PortIndex "Index [-]"; PortWidth "Port Dimensions [-]"; TimeStep "Upload Period [s]"
      mask help: Sends signal(s) from Simulink to the Sensor World
      mask Help:
      | Sends signal(s) from Simulink to the Sensor World.
      | 
      | - Port Name [-]: specifies the name of the output terminal of the Simulink block.
      | - Index [-]: specifies the start position in the output vector sent to the Sensor World
      | - Port dimensions [-]: specifies the width (number of scalars) of the output port
      | - Upload period [s]: specifies the upload period (in seconds) of the port.
      mask Display: port_label('input', 1, PortName)
  visu_IndicatorLightsRight_0 : Subsystem  mask(ToSensorWorld){PortName=visu_IndicatorLightsRight_0, PortIndex=236, PortWidth=16, TimeStep=1/100}
      mask prompts: PortName "Port Name [-]"; PortIndex "Index [-]"; PortWidth "Port Dimensions [-]"; TimeStep "Upload Period [s]"
      mask help: Sends signal(s) from Simulink to the Sensor World
      mask Help:
      | Sends signal(s) from Simulink to the Sensor World.
      | 
      | - Port Name [-]: specifies the name of the output terminal of the Simulink block.
      | - Index [-]: specifies the start position in the output vector sent to the Sensor World
      | - Port dimensions [-]: specifies the width (number of scalars) of the output port
      | - Upload period [s]: specifies the upload period (in seconds) of the port.
      mask Display: port_label('input', 1, PortName)
  visu_MainLightFrontLeft_0 : Subsystem  mask(ToSensorWorld){PortName=visu_MainLightFrontLeft_0, PortIndex=348, PortWidth=16, TimeStep=1/100}
      mask prompts: PortName "Port Name [-]"; PortIndex "Index [-]"; PortWidth "Port Dimensions [-]"; TimeStep "Upload Period [s]"
      mask help: Sends signal(s) from Simulink to the Sensor World
      mask Help:
      | Sends signal(s) from Simulink to the Sensor World.
      | 
      | - Port Name [-]: specifies the name of the output terminal of the Simulink block.
      | - Index [-]: specifies the start position in the output vector sent to the Sensor World
      | - Port dimensions [-]: specifies the width (number of scalars) of the output port
      | - Upload period [s]: specifies the upload period (in seconds) of the port.
      mask Display: port_label('input', 1, PortName)
  visu_MainLightFrontRight_0 : Subsystem  mask(ToSensorWorld){PortName=visu_MainLightFrontRight_0, PortIndex=364, PortWidth=16, TimeStep=1/100}
      mask prompts: PortName "Port Name [-]"; PortIndex "Index [-]"; PortWidth "Port Dimensions [-]"; TimeStep "Upload Period [s]"
      mask help: Sends signal(s) from Simulink to the Sensor World
      mask Help:
      | Sends signal(s) from Simulink to the Sensor World.
      | 
      | - Port Name [-]: specifies the name of the output terminal of the Simulink block.
      | - Index [-]: specifies the start position in the output vector sent to the Sensor World
      | - Port dimensions [-]: specifies the width (number of scalars) of the output port
      | - Upload period [s]: specifies the upload period (in seconds) of the port.
      mask Display: port_label('input', 1, PortName)
  visu_MainLightRearLeft_0 : Subsystem  mask(ToSensorWorld){PortName=visu_MainLightRearLeft_0, PortIndex=316, PortWidth=16, TimeStep=1/100}
      mask prompts: PortName "Port Name [-]"; PortIndex "Index [-]"; PortWidth "Port Dimensions [-]"; TimeStep "Upload Period [s]"
      mask help: Sends signal(s) from Simulink to the Sensor World
      mask Help:
      | Sends signal(s) from Simulink to the Sensor World.
      | 
      | - Port Name [-]: specifies the name of the output terminal of the Simulink block.
      | - Index [-]: specifies the start position in the output vector sent to the Sensor World
      | - Port dimensions [-]: specifies the width (number of scalars) of the output port
      | - Upload period [s]: specifies the upload period (in seconds) of the port.
      mask Display: port_label('input', 1, PortName)
  visu_MainLightRearRight_0 : Subsystem  mask(ToSensorWorld){PortName=visu_MainLightRearRight_0, PortIndex=332, PortWidth=16, TimeStep=1/100}
      mask prompts: PortName "Port Name [-]"; PortIndex "Index [-]"; PortWidth "Port Dimensions [-]"; TimeStep "Upload Period [s]"
      mask help: Sends signal(s) from Simulink to the Sensor World
      mask Help:
      | Sends signal(s) from Simulink to the Sensor World.
      | 
      | - Port Name [-]: specifies the name of the output terminal of the Simulink block.
      | - Index [-]: specifies the start position in the output vector sent to the Sensor World
      | - Port dimensions [-]: specifies the width (number of scalars) of the output port
      | - Upload period [s]: specifies the upload period (in seconds) of the port.
      mask Display: port_label('input', 1, PortName)
  wires:
  Lights/1 -> SubDemuxVisuLightSource/1
  SubDemuxVisuLightSource/1 -> Saturation1/1
  Saturation1/1 -> conv_IndicatorLightsLeft/1(on_off)
  conv_IndicatorLightsLeft/1(RGBcolor) -> visu_IndicatorLightsLeft_0/1(Y)
  SubDemuxVisuLightSource/2 -> Saturation2/1
  Saturation2/1 -> conv_IndicatorLightsRight/1(on_off)
  conv_IndicatorLightsRight/1(RGBcolor) -> visu_IndicatorLightsRight_0/1(Y)
  SubDemuxVisuLightSource/3 -> Saturation3/1
  Saturation3/1 -> conv_FogLightRearLeft/1(on_off)
  conv_FogLightRearLeft/1(RGBcolor) -> visu_FogLightRearLeft_0/1(Y)
  SubDemuxVisuLightSource/4 -> Saturation4/1
  Saturation4/1 -> conv_FogLightRearRight/1(on_off)
  conv_FogLightRearRight/1(RGBcolor) -> visu_FogLightRearRight_0/1(Y)
  SubDemuxVisuLightSource/5 -> Saturation5/1
  Saturation5/1 -> conv_FogLightFrontLeft/1(on_off)
  conv_FogLightFrontLeft/1(RGBcolor) -> visu_FogLightFrontLeft_0/1(Y)
  SubDemuxVisuLightSource/6 -> Saturation6/1
  Saturation6/1 -> conv_FogLightFrontRight/1(on_off)
  conv_FogLightFrontRight/1(RGBcolor) -> visu_FogLightFrontRight_0/1(Y)
  SubDemuxVisuLightSource/7 -> Saturation7/1
  Saturation7/1 -> conv_MainLightRearLeft/1(on_off)
  conv_MainLightRearLeft/1(RGBcolor) -> visu_MainLightRearLeft_0/1(Y)
  SubDemuxVisuLightSource/8 -> Saturation8/1
  Saturation8/1 -> conv_MainLightRearRight/1(on_off)
  conv_MainLightRearRight/1(RGBcolor) -> visu_MainLightRearRight_0/1(Y)
  SubDemuxVisuLightSource/9 -> Saturation9/1
  Saturation9/1 -> conv_MainLightFrontLeft/1(on_off)
  conv_MainLightFrontLeft/1(RGBcolor) -> visu_MainLightFrontLeft_0/1(Y)
  SubDemuxVisuLightSource/10 -> Saturation10/1
  Saturation10/1 -> conv_MainLightFrontRight/1(on_off)
  conv_MainLightFrontRight/1(RGBcolor) -> visu_MainLightFrontRight_0/1(Y)
  SubDemuxVisuLightSource/11 -> Saturation11/1
  Saturation11/1 -> conv_BrakeLightLeft/1(on_off)
  conv_BrakeLightLeft/1(RGBcolor) -> visu_BrakeLightLeft_0/1(Y)
  SubDemuxVisuLightSource/12 -> Saturation12/1
  Saturation12/1 -> conv_BrakeLightRight/1(on_off)
  conv_BrakeLightRight/1(RGBcolor) -> visu_BrakeLightRight_0/1(Y)
